simulink model "ClimaTotFlowForHvacRe"  (R2022b)
settings: Solver=FixedStepDiscrete  StartTime=0.0  StopTime=10.0  FixedStep=0.2  RelTol=1e-3
settings Model: Creator=blue.ge  LastModifiedBy=blue.ge
settings DataIOCC: SaveFormat=StructureWithTime
settings OptimizationCC: DefaultParameterBehavior=Inlined  EnhancedBackFolding=on  PassReuseOutputArgsAs=Individual arguments  ZeroExternalMemoryAtStartup=off  ZeroInternalMemoryAtStartup=off  OptimizeBlockOrder=speed  DifferentSizesBufferReuse=on  OptimizationCustomize=off  EfficientTunableParamExpr=on
settings DebuggingCC: ParameterTunabilityLossMsg=error
settings ModelReferenceCC: ModelReferenceNumInstancesAllowed=Single
settings SFSimCC: MATLABDynamicMemAlloc=off
settings RTWCC: Description=AUTOSAR  SystemTargetFile=autosar.tlc  GenCodeOnly=on  TemplateMakefile=ert_default_tmf
settings CodeAppCC: ForceParamTrailComments=on  IgnoreCustomStorageClasses=off  OperatorAnnotations=on  SimulinkDataObjDesc=on  SFDataObjDesc=on  InsertBlockDesc=on
settings STFCustomTargetCC: TargetLangStandard=C99 (ISO)  MultiwordLength=256  ParMdlRefBuildCompliant=on  CompOptLevelCompliant=on  IncludeMdlTerminateFcn=off  CombineOutputUpdateFcns=on  SuppressErrorStatus=on  ERTCustomFileBanners=on  SupportNonFinite=off  SupportComplex=off  SupportContinuousTime=off  SupportNonInlinedSFcns=off  RemoveResetFunc=on  MATLABClassNameForMDSCustomization=Simulink.SoftwareTarget.ERTCustomization  AutosarCompliant=on  UseToolchainInfoCompliant=on  LookupTableObjectStructAxisOrder=2,1,3,4,...  LUTObjectStructOrderEvenSpacing=Size,Table,Breakpoints  SystemTargetFile=autosar.tlc
settings ERTTargetCC: TargetLangStandard=C99 (ISO)  ConcurrentExecutionCompliant=off  IncludeMdlTerminateFcn=off  SuppressErrorStatus=on  SupportNonFinite=off  SupportComplex=off  ModelStepFunctionPrototypeControlCompliant=off  CPPClassGenCompliant=off  AutosarCompliant=on  LookupTableObjectStructAxisOrder=2,1,3,4,...  LUTObjectStructOrderEvenSpacing=Size,Table,Breakpoints  InlinedParameterPlacement=NonHierarchical  ERTCustomFileTemplate=example_file_process.tlc

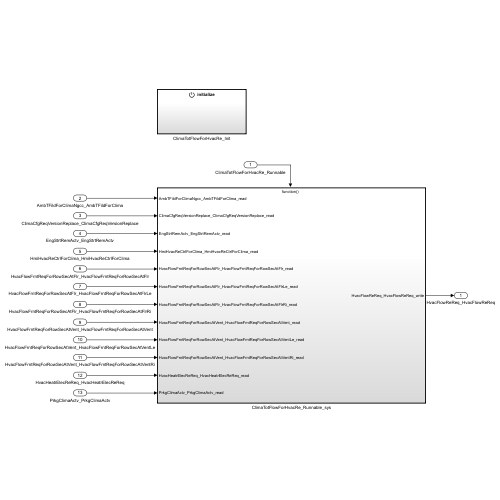
[thumbnail: Simulink's stored preview of the top-level diagram]
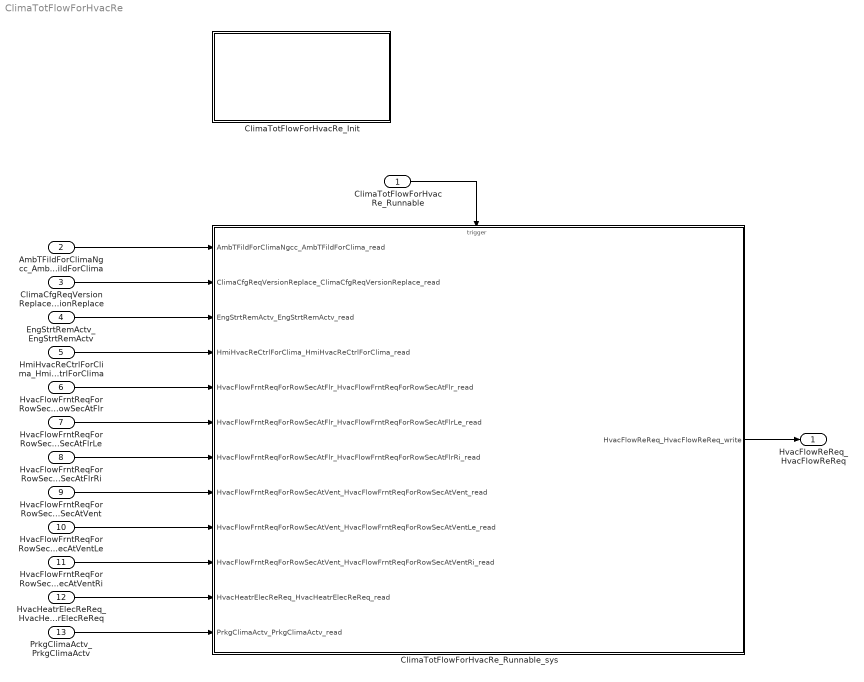
== system ClimaTotFlowForHvacRe ==
  ClimaTotFlowForHvacRe_Runnable : Inport  SampleTime=0.2  OutputFunctionCall=on
  AmbTFildForClimaNgcc_AmbTFildForClima : Inport  Port=2  OutDataTypeStr=T30  PortDimensions=1
  ClimaCfgReqVersionReplace_ClimaCfgReqVersionReplace : Inport  Port=3  OutDataTypeStr=UInt8  PortDimensions=1
  EngStrtRemActv_EngStrtRemActv : Inport  Port=4  OutDataTypeStr=Boolean  PortDimensions=1
  HmiHvacReCtrlForClima_HmiHvacReCtrlForClima : Inport  Port=5  OutDataTypeStr=Enum: HmiHvacReCtrl  PortDimensions=1
  HvacFlowFrntReqForRowSecAtFlr_HvacFlowFrntReqForRowSecAtFlr : Inport  Port=6  OutDataTypeStr=AirFlow30  PortDimensions=1
  HvacFlowFrntReqForRowSecAtFlr_HvacFlowFrntReqForRowSecAtFlrLe : Inport  Port=7  OutDataTypeStr=AirFlow30  PortDimensions=1
  HvacFlowFrntReqForRowSecAtFlr_HvacFlowFrntReqForRowSecAtFlrRi : Inport  Port=8  OutDataTypeStr=AirFlow30  PortDimensions=1
  HvacFlowFrntReqForRowSecAtVent_HvacFlowFrntReqForRowSecAtVent : Inport  Port=9  OutDataTypeStr=AirFlow30  PortDimensions=1
  HvacFlowFrntReqForRowSecAtVent_HvacFlowFrntReqForRowSecAtVentLe : Inport  Port=10  OutDataTypeStr=AirFlow30  PortDimensions=1
  HvacFlowFrntReqForRowSecAtVent_HvacFlowFrntReqForRowSecAtVentRi : Inport  Port=11  OutDataTypeStr=AirFlow30  PortDimensions=1
  HvacHeatrElecReReq_HvacHeatrElecReReq : Inport  Port=12  OutDataTypeStr=Enum: OnOff1  PortDimensions=1
  PrkgClimaActv_PrkgClimaActv : Inport  Port=13  OutDataTypeStr=Enum: PrkgClimaActv  PortDimensions=1
  ClimaTotFlowForHvacRe_Init : Subsystem
  ClimaTotFlowForHvacRe_Runnable_sys : Subsystem
  HvacFlowReReq_HvacFlowReReq : Outport  OutDataTypeStr=AirFlow30  PortDimensions=1
  wires:
  AmbTFildForClimaNgcc_AmbTFildForClima/1 -> ClimaTotFlowForHvacRe_Runnable_sys/1(AmbTFildForClimaNgcc_AmbTFildForClima_read)
  ClimaCfgReqVersionReplace_ClimaCfgReqVersionReplace/1 -> ClimaTotFlowForHvacRe_Runnable_sys/2(ClimaCfgReqVersionReplace_ClimaCfgReqVersionReplace_read)
  EngStrtRemActv_EngStrtRemActv/1 -> ClimaTotFlowForHvacRe_Runnable_sys/3(EngStrtRemActv_EngStrtRemActv_read)
  HmiHvacReCtrlForClima_HmiHvacReCtrlForClima/1 -> ClimaTotFlowForHvacRe_Runnable_sys/4(HmiHvacReCtrlForClima_HmiHvacReCtrlForClima_read)
  HvacFlowFrntReqForRowSecAtFlr_HvacFlowFrntReqForRowSecAtFlr/1 -> ClimaTotFlowForHvacRe_Runnable_sys/5(HvacFlowFrntReqForRowSecAtFlr_HvacFlowFrntReqForRowSecAtFlr_read)
  HvacFlowFrntReqForRowSecAtFlr_HvacFlowFrntReqForRowSecAtFlrLe/1 -> ClimaTotFlowForHvacRe_Runnable_sys/6(HvacFlowFrntReqForRowSecAtFlr_HvacFlowFrntReqForRowSecAtFlrLe_read)
  HvacFlowFrntReqForRowSecAtFlr_HvacFlowFrntReqForRowSecAtFlrRi/1 -> ClimaTotFlowForHvacRe_Runnable_sys/7(HvacFlowFrntReqForRowSecAtFlr_HvacFlowFrntReqForRowSecAtFlrRi_read)
  HvacFlowFrntReqForRowSecAtVent_HvacFlowFrntReqForRowSecAtVent/1 -> ClimaTotFlowForHvacRe_Runnable_sys/8(HvacFlowFrntReqForRowSecAtVent_HvacFlowFrntReqForRowSecAtVent_read)
  HvacFlowFrntReqForRowSecAtVent_HvacFlowFrntReqForRowSecAtVentLe/1 -> ClimaTotFlowForHvacRe_Runnable_sys/9(HvacFlowFrntReqForRowSecAtVent_HvacFlowFrntReqForRowSecAtVentLe_read)
  HvacFlowFrntReqForRowSecAtVent_HvacFlowFrntReqForRowSecAtVentRi/1 -> ClimaTotFlowForHvacRe_Runnable_sys/10(HvacFlowFrntReqForRowSecAtVent_HvacFlowFrntReqForRowSecAtVentRi_read)
  HvacHeatrElecReReq_HvacHeatrElecReReq/1 -> ClimaTotFlowForHvacRe_Runnable_sys/11(HvacHeatrElecReReq_HvacHeatrElecReReq_read)
  PrkgClimaActv_PrkgClimaActv/1 -> ClimaTotFlowForHvacRe_Runnable_sys/12(PrkgClimaActv_PrkgClimaActv_read)
  ClimaTotFlowForHvacRe_Runnable_sys/1(HvacFlowReReq_HvacFlowReReq_write) -> HvacFlowReReq_HvacFlowReReq/1
  ClimaTotFlowForHvacRe_Runnable/1 -> ClimaTotFlowForHvacRe_Runnable_sys/Trigger

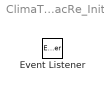
== system ClimaTotFlowForHvacRe/ClimaTotFlowForHvacRe_Init ==
  Event Listener : EventListener  EventName=reset

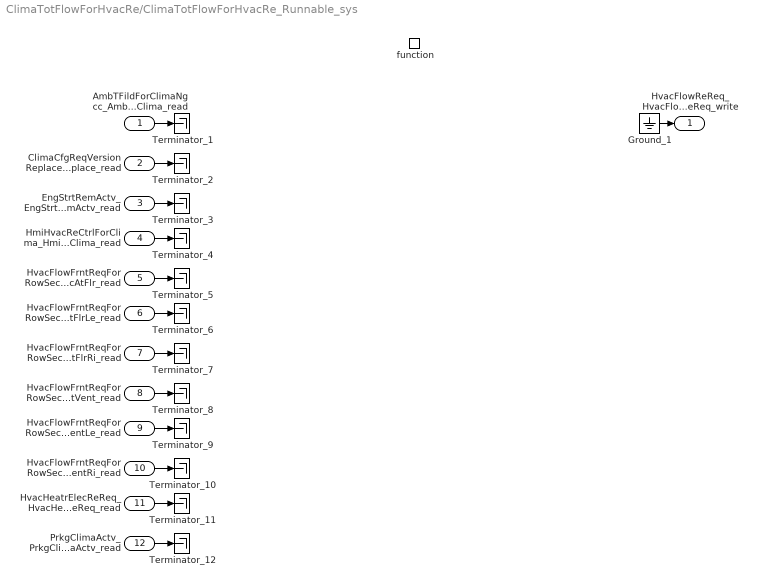
== system ClimaTotFlowForHvacRe/ClimaTotFlowForHvacRe_Runnable_sys ==
  AmbTFildForClimaNgcc_AmbTFildForClima_read : Inport
  ClimaCfgReqVersionReplace_ClimaCfgReqVersionReplace_read : Inport  Port=2
  EngStrtRemActv_EngStrtRemActv_read : Inport  Port=3
  HmiHvacReCtrlForClima_HmiHvacReCtrlForClima_read : Inport  Port=4
  HvacFlowFrntReqForRowSecAtFlr_HvacFlowFrntReqForRowSecAtFlr_read : Inport  Port=5
  HvacFlowFrntReqForRowSecAtFlr_HvacFlowFrntReqForRowSecAtFlrLe_read : Inport  Port=6
  HvacFlowFrntReqForRowSecAtFlr_HvacFlowFrntReqForRowSecAtFlrRi_read : Inport  Port=7
  HvacFlowFrntReqForRowSecAtVent_HvacFlowFrntReqForRowSecAtVent_read : Inport  Port=8
  HvacFlowFrntReqForRowSecAtVent_HvacFlowFrntReqForRowSecAtVentLe_read : Inport  Port=9
  HvacFlowFrntReqForRowSecAtVent_HvacFlowFrntReqForRowSecAtVentRi_read : Inport  Port=10
  HvacHeatrElecReReq_HvacHeatrElecReReq_read : Inport  Port=11
  PrkgClimaActv_PrkgClimaActv_read : Inport  Port=12
  function : TriggerPort  TriggerType=function-call  VariantControl=(inherit)  SampleTimeType=periodic  SampleTime=-1
  Ground_1 : Ground
  Terminator_{1..12} (12 alike) : Terminator
  HvacFlowReReq_HvacFlowReReq_write : Outport
      settings: EnsureOutportIsVirtual=on
  wires:
  AmbTFildForClimaNgcc_AmbTFildForClima_read/1 -> Terminator_1/1
  ClimaCfgReqVersionReplace_ClimaCfgReqVersionReplace_read/1 -> Terminator_2/1
  EngStrtRemActv_EngStrtRemActv_read/1 -> Terminator_3/1
  HmiHvacReCtrlForClima_HmiHvacReCtrlForClima_read/1 -> Terminator_4/1
  HvacFlowFrntReqForRowSecAtFlr_HvacFlowFrntReqForRowSecAtFlr_read/1 -> Terminator_5/1
  HvacFlowFrntReqForRowSecAtFlr_HvacFlowFrntReqForRowSecAtFlrLe_read/1 -> Terminator_6/1
  HvacFlowFrntReqForRowSecAtFlr_HvacFlowFrntReqForRowSecAtFlrRi_read/1 -> Terminator_7/1
  HvacFlowFrntReqForRowSecAtVent_HvacFlowFrntReqForRowSecAtVent_read/1 -> Terminator_8/1
  HvacFlowFrntReqForRowSecAtVent_HvacFlowFrntReqForRowSecAtVentLe_read/1 -> Terminator_9/1
  HvacFlowFrntReqForRowSecAtVent_HvacFlowFrntReqForRowSecAtVentRi_read/1 -> Terminator_10/1
  HvacHeatrElecReReq_HvacHeatrElecReReq_read/1 -> Terminator_11/1
  PrkgClimaActv_PrkgClimaActv_read/1 -> Terminator_12/1
  Ground_1/1 -> HvacFlowReReq_HvacFlowReReq_write/1
